simulink model "Lab2_simulink_plc"  (R2023b)
settings: Solver=FixedStepAuto  StartTime=0.0  StopTime=inf  RelTol=1e-3
InitFcn:
  | d13_init.PRE = 50;
undefined symbols (not defined in the file or in its folder): BOOL, DINT, OTL, slplc
libraries: arduinolib x9
(part 4 of 31)

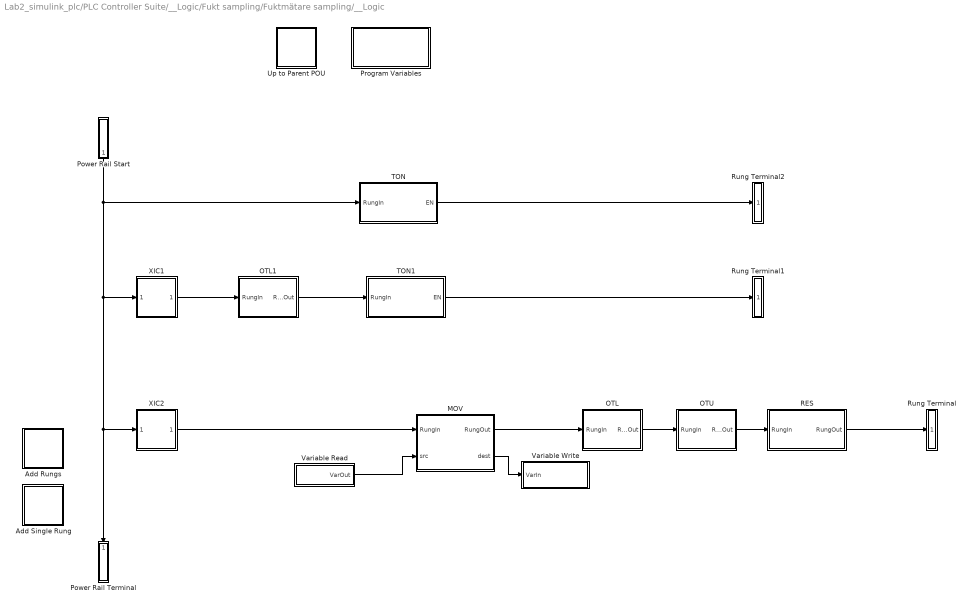
== system Lab2_simulink_plc/PLC Controller Suite/__Logic/Fukt sampling/Fuktmätare sampling/__Logic ==
  Add Rungs : Subsystem  DisableCoverage=on  TreatAsAtomicUnit=on  mask{PLCRungNumberToAdd=2, PLCBlockType=AddRungs}
      mask prompts: PLCRungNumberToAdd "Rung Number:"
      mask Help: web([docroot '/plccoder/ref/addrungs' '.html']);
      mask DialogControl PLCAddRungs Prompt: Add Rungs
      mask DialogControl PLCRungAddButton Prompt: Add
      mask DialogControl PLCRungAddButton Callback: slplc.callbacks.addLadderRungs(gcb);
      mask Display: disp(sprintf(['Add' newline 'Rungs']));
  Add Single Rung : Subsystem  DisableCoverage=on  TreatAsAtomicUnit=on  mask{PLCRungNumberToAdd=1, PLCBlockType=AddSingleRung}
      settings: OpenFcn=slplc.callbacks.addLadderRungs(gcb);
      mask Help: web([docroot '/plccoder/ref/addsinglerung' '.html']);
      mask DialogControl PLCAddRung Prompt: Add Single Rung
      mask Display: disp(sprintf(['Add' newline '1 Rung']));
  MOV : Subsystem  Priority=10  TreatAsAtomicUnit=on  mask{PLCBlockType=MOV, PLCPOUType=stdFC}
      mask Help: web([docroot '/plccoder/ref/mov' '.html']);
      mask DialogControl PLCBlockTitle Prompt: Move (MOV)
      mask DialogControl PLCBlockDesc Prompt: Move a copy of the Source to the Destination. The Source remains unchanged.
      mask Display: disp('MOV');
  OTL : Subsystem  Priority=12  TreatAsAtomicUnit=on  mask{PLCOperandTag=fukt_polling, PLCBlockType=OTL, PLCPOUType=Coil, PLCOperandExpression=xxx_PLC_VAR_fukt_polling}
      mask prompts: PLCOperandTag "Operand Tag:"
      mask init:
      | slplc.callbacks.initInstruction(gcb);
      settings: AttributesFormatString=%<PLCOperandTag>
      mask Help: web([docroot '/plccoder/ref/otl' '.html']);
      mask DialogControl PLCBlockTitle Prompt: Output Latch (OTL)
      mask Display: disp('L');
  OTL1 : Subsystem  Priority=5  TreatAsAtomicUnit=on  mask{PLCOperandTag=fukt_power, PLCBlockType=OTL, PLCPOUType=Coil, PLCOperandExpression=xxx_PLC_VAR_fukt_power}
      mask prompts: PLCOperandTag "Operand Tag:"
      mask init:
      | slplc.callbacks.initInstruction(gcb);
      settings: AttributesFormatString=%<PLCOperandTag>
      mask Help: web([docroot '/plccoder/ref/otl' '.html']);
      mask DialogControl PLCBlockTitle Prompt: Output Latch (OTL)
      mask Display: disp('L');
  OTU : Subsystem  Priority=13  TreatAsAtomicUnit=on  mask{PLCOperandTag=fukt_power, PLCBlockType=OTU, PLCPOUType=Coil, PLCOperandExpression=xxx_PLC_VAR_fukt_power}
      mask prompts: PLCOperandTag "Operand Tag:"
      mask init:
      | slplc.callbacks.initInstruction(gcb);
      settings: AttributesFormatString=%<PLCOperandTag>
      mask Help: web([docroot '/plccoder/ref/otu' '.html']);
      mask DialogControl PLCBlockTitle Prompt: Output Unlatch (OTU)
      mask Display: disp('U');
  Power Rail Start : Subsystem  Priority=1  DisableCoverage=on  TreatAsAtomicUnit=on  mask{PLCBlockType=PowerRailStart}
      mask Help: web([docroot '/plccoder/ref/powerrailstart' '.html']);
      mask DialogControl PLCBlockTitle Prompt: Power Rail Start
      mask DialogControl PLCBlockDesc Prompt: This is the ladder diagram left power rail start block.
  Power Rail Terminal : Subsystem  DisableCoverage=on  TreatAsAtomicUnit=on  mask{PLCBlockType=PowerRailTerminal}
      mask Help: web([docroot '/plccoder/ref/powerrailterminal' '.html']);
      mask DialogControl PLCBlockTitle Prompt: Power Rail Terminal
      mask DialogControl PLCBlockDesc Prompt: This is the ladder diagram left power rail terminal block.
  Program Variables : Subsystem  Tag=ProgramVariableSS  DisableCoverage=on  TreatAsAtomicUnit=on  mask{PLCVarFukt_periodicityName=Fukt_periodicity, PLCVarFukt_periodicityScope=Local, PLCVarFukt_periodicityPortType=Hidden, PLCVarFukt_periodicityPortIndex=6, PLCVarFukt_periodicityDataType=Bus: TIMER, PLCVarFukt_periodicitySize=1, PLCVarFukt_periodicityInitialValue=fukt_period, PLCVarFukt_periodicityToDelete=off, PLCVard13_waitName=d13_wait, PLCVard13_waitScope=Local, PLCVard13_waitPortType=Hidden, PLCVard13_waitPortIndex=6, PLCVard13_waitDataType=Bus: TIMER, PLCVard13_waitSize=1, PLCVard13_waitInitialValue=d13_period, PLCVard13_waitToDelete=off, PLCVarFukt_valueName=Fukt_value, PLCVarFukt_valueScope=External, PLCVarFukt_valuePortType=Hidden, PLCVarFukt_valuePortIndex=6, PLCVarFukt_valueDataType=DINT, PLCVarFukt_valueSize=-1, PLCVarFukt_valueInitialValue=0, PLCVarFukt_valueToDelete=off, PLCVarFukt_value_copyName=Fukt_value_copy, PLCVarFukt_value_copyScope=External, PLCVarFukt_value_copyPortType=Hidden, PLCVarFukt_value_copyPortIndex=6, PLCVarFukt_value_copyDataType=DINT, PLCVarFukt_value_copySize=-1, PLCVarFukt_value_copyInitialValue=0, PLCVarFukt_value_copyToDelete=off, PLCVarfukt_pollingName=fukt_polling, PLCVarfukt_pollingScope=External, PLCVarfukt_pollingPortType=Hidden, PLCVarfukt_pollingPortIndex=6, PLCVarfukt_pollingDataType=DINT, PLCVarfukt_pollingSize=-1, PLCVarfukt_pollingInitialValue=0, PLCVarfukt_pollingToDelete=off, PLCVarfukt_powerName=fukt_power, PLCVarfukt_powerScope=External, PLCVarfukt_powerPortType=Hidden, PLCVarfukt_powerPortIndex=6, PLCVarfukt_powerDataType=DINT, PLCVarfukt_powerSize=-1, PLCVarfukt_powerInitialValue=0, PLCVarfukt_powerToDelete=off}
      mask init:
      | slplc.callbacks.initPOUVarSS(gcb);
      settings: OpenFcn=slplc.callbacks.openProgramVariableSS(gcb);
      mask Help: web([docroot '/plccoder/ref/programvariables' '.html']);
      mask TypeOptions PLCVarFukt_periodicityScope: Local | External
      mask Callback PLCVarFukt_periodicityScope: slplc.callbacks.updatePOUVarSS(gcb);
      mask TypeOptions PLCVarFukt_periodicityPortType: Hidden | Inport | Outport
      mask Callback PLCVarFukt_periodicityPortType: slplc.callbacks.updatePOUVarSS(gcb);
      mask TypeOptions PLCVarFukt_periodicityPortIndex: 1 | 2 | 3 | 4 | 5 | 6
      mask TypeOptions PLCVard13_waitScope: Local | External
      mask Callback PLCVard13_waitScope: slplc.callbacks.updatePOUVarSS(gcb);
      mask TypeOptions PLCVard13_waitPortType: Hidden | Inport | Outport
      mask Callback PLCVard13_waitPortType: slplc.callbacks.updatePOUVarSS(gcb);
      mask TypeOptions PLCVard13_waitPortIndex: 1 | 2 | 3 | 4 | 5 | 6
      mask TypeOptions PLCVarFukt_valueScope: Local | External
      mask Callback PLCVarFukt_valueScope: slplc.callbacks.updatePOUVarSS(gcb);
      mask TypeOptions PLCVarFukt_valuePortType: Hidden | Inport | Outport
      mask Callback PLCVarFukt_valuePortType: slplc.callbacks.updatePOUVarSS(gcb);
      mask TypeOptions PLCVarFukt_valuePortIndex: 1 | 2 | 3 | 4 | 5 | 6
      mask TypeOptions PLCVarFukt_value_copyScope: Local | External
      mask Callback PLCVarFukt_value_copyScope: slplc.callbacks.updatePOUVarSS(gcb);
      mask TypeOptions PLCVarFukt_value_copyPortType: Hidden | Inport | Outport
      mask Callback PLCVarFukt_value_copyPortType: slplc.callbacks.updatePOUVarSS(gcb);
      mask TypeOptions PLCVarFukt_value_copyPortIndex: 1 | 2 | 3 | 4 | 5 | 6
      mask TypeOptions PLCVarfukt_pollingScope: Local | External
      mask Callback PLCVarfukt_pollingScope: slplc.callbacks.updatePOUVarSS(gcb);
      mask TypeOptions PLCVarfukt_pollingPortType: Hidden | Inport | Outport
      mask Callback PLCVarfukt_pollingPortType: slplc.callbacks.updatePOUVarSS(gcb);
      mask TypeOptions PLCVarfukt_pollingPortIndex: 1 | 2 | 3 | 4 | 5 | 6
      mask TypeOptions PLCVarfukt_powerScope: Local | External
      mask Callback PLCVarfukt_powerScope: slplc.callbacks.updatePOUVarSS(gcb);
      mask TypeOptions PLCVarfukt_powerPortType: Hidden | Inport | Outport
      mask Callback PLCVarfukt_powerPortType: slplc.callbacks.updatePOUVarSS(gcb);
      mask TypeOptions PLCVarfukt_powerPortIndex: 1 | 2 | 3 | 4 | 5 | 6
      mask DialogControl PLCBlockTitle Prompt: Program Variable Spreadsheet
      mask DialogControl PLCVarListHeaderName Prompt: Name
      mask DialogControl PLCVarListHeaderScope Prompt: Scope
      mask DialogControl PLCVarListHeaderDataType Prompt: Data Type
      mask DialogControl PLCVarListHeaderSize Prompt: Size
      mask DialogControl PLCVarListHeaderInitialValue Prompt: Initial Value
      mask DialogControl PLCVarListHeaderToDelete Prompt: Delete
  RES : Subsystem  Tag=B  Priority=14  TreatAsAtomicUnit=on  mask{PLCOperandTag=Fukt_periodicity, PLCTagDataType=TIMER, PLCBlockType=RES, PLCPOUType=stdFC, PLCOperandExpression=xxx_PLC_VAR_Fukt_periodicity}
      mask prompts: PLCOperandTag "Operand Tag To Reset:"; PLCTagDataType "Tag Structure:"
      mask init:
      | block = gcb;
      | maskObj = Simulink.Mask.get(block);
      | maskObj.Display = sprintf('disp([''%s''  newline newline ''%s'']);', 'RES', PLCOperandTag);
      | slplc.callbacks.initResetBlock(block);
      | slplc.callbacks.initInstruction(block);
      settings: AttributesFormatString=%<PLCBlockType>: %<PLCOperandTag>
      mask Help: web([docroot '/plccoder/ref/res' '.html']);
      mask TypeOptions PLCTagDataType: TIMER | COUNTER | CONTROL
      mask DialogControl PLCBlockTitle Prompt: Reset TIMER, COUNTER, or CONTROL structure (RES)
      mask Display: disp(['RES'  newline newline 'Fukt_periodicity']);
  Rung Terminal : Subsystem  Priority=15  DisableCoverage=on  TreatAsAtomicUnit=on  mask{PLCRungTerminalIndex=3, PLCBlockType=RungTerminal}
      settings: AttributesFormatString=Rung %<PLCRungTerminalIndex>
      mask Help: web([docroot '/plccoder/ref/rungterminal' '.html']);
      mask DialogControl PLCBlockTitle Prompt: Rung Terminal
      mask DialogControl PLCBlockDescText Prompt: This is the ladder diagram rung terminal.
  Rung Terminal1 : Subsystem  Priority=7  DisableCoverage=on  TreatAsAtomicUnit=on  mask{PLCRungTerminalIndex=2, PLCBlockType=RungTerminal}
      settings: AttributesFormatString=Rung %<PLCRungTerminalIndex>
      mask Help: web([docroot '/plccoder/ref/rungterminal' '.html']);
      mask DialogControl PLCBlockTitle Prompt: Rung Terminal
      mask DialogControl PLCBlockDescText Prompt: This is the ladder diagram rung terminal.
  Rung Terminal2 : Subsystem  Priority=3  DisableCoverage=on  TreatAsAtomicUnit=on  mask{PLCRungTerminalIndex=1, PLCBlockType=RungTerminal}
      settings: AttributesFormatString=Rung %<PLCRungTerminalIndex>
      mask Help: web([docroot '/plccoder/ref/rungterminal' '.html']);
      mask DialogControl PLCBlockTitle Prompt: Rung Terminal
      mask DialogControl PLCBlockDescText Prompt: This is the ladder diagram rung terminal.
  TON : Subsystem  Priority=2  TreatAsAtomicUnit=on  mask{PLCOperandTag=Fukt_periodicity, PLCTimerSampleTime=0.2, PLCBlockType=TON, PLCPOUType=stdFB, PLCOperandAccess=ReadWrite, PLCOperandExpression=xxx_PLC_VAR_Fukt_periodicity}
      mask prompts: PLCOperandTag "Timer Tag:"; PLCTimerSampleTime "Timer Sample Time:"
      mask init:
      | block = gcb;
      | maskObj = Simulink.Mask.get(block);
      | maskObj.Display = sprintf('disp([''%s'' newline newline ''%s'']);', PLCBlockType, PLCOperandTag);
      | slplc.callbacks.initInstruction(block);
      settings: AttributesFormatString=%<PLCBlockType>: %<PLCOperandTag>
      mask Help: web([docroot '/plccoder/ref/ton' '.html']);
      mask DialogControl PLCBlockTitle Prompt: Timer On Delay (TON)
      mask Display: disp(['TON' newline newline 'Fukt_periodicity']);
  TON1 : Subsystem  Priority=6  TreatAsAtomicUnit=on  mask{PLCOperandTag=d13_wait, PLCTimerSampleTime=0.2, PLCBlockType=TON, PLCPOUType=stdFB, PLCOperandAccess=ReadWrite, PLCOperandExpression=xxx_PLC_VAR_d13_wait}
      mask prompts: PLCOperandTag "Timer Tag:"; PLCTimerSampleTime "Timer Sample Time:"
      mask init:
      | block = gcb;
      | maskObj = Simulink.Mask.get(block);
      | maskObj.Display = sprintf('disp([''%s'' newline newline ''%s'']);', PLCBlockType, PLCOperandTag);
      | slplc.callbacks.initInstruction(block);
      settings: AttributesFormatString=%<PLCBlockType>: %<PLCOperandTag>
      mask Help: web([docroot '/plccoder/ref/ton' '.html']);
      mask DialogControl PLCBlockTitle Prompt: Timer On Delay (TON)
      mask Display: disp(['TON' newline newline 'd13_wait']);
  Up to Parent POU : Subsystem  DisableCoverage=on  TreatAsAtomicUnit=on  mask{PLCBlockType=OpenParentPOU}
      settings: OpenFcn=slplc.callbacks.openParentPOUBlock(gcb);
      mask Help: web([docroot '/plccoder/ref/uptoparentpou' '.html']);
      mask DialogControl PLCBlockTitle Prompt: Add Single Rung
  Variable Read : Subsystem  Priority=9  TreatAsAtomicUnit=on  mask{PLCOperandTag=Fukt_value, PLCBlockType=VariableRead, PLCOperandAccess=Read, PLCPOUType=VariableRead, PLCOperandExpression=xxx_PLC_VAR_Fukt_value}
      mask prompts: PLCOperandTag "Data Tag:"
      mask init:
      | block = gcb;
      | maskObj = Simulink.Mask.get(block);
      | maskObj.Display = sprintf('disp(''%s'');', PLCOperandTag);
      | slplc.callbacks.initInstruction(block);
      mask Help: web([docroot '/plccoder/ref/variableread' '.html']);
      mask DialogControl PLCBlockTitle Prompt: Data Tag Read
      mask Display: disp('Fukt_value');
  Variable Write : Subsystem  Priority=11  TreatAsAtomicUnit=on  mask{PLCOperandTag=Fukt_value_copy, PLCBlockType=VariableWrite, PLCOperandAccess=Write, PLCPOUType=VariableWrite, PLCOperandExpression=xxx_PLC_VAR_Fukt_value_copy}
      mask prompts: PLCOperandTag "Data Tag:"
      mask init: same as Lab2_simulink_plc/PLC Controller Suite/__Logic/Fukt logic/Program/__Logic/Variable Write
      mask Help: web([docroot '/plccoder/ref/variablewrite' '.html']);
      mask DialogControl PLCBlockTitle Prompt: Data Tag Write
      mask Display: disp('Fukt_value_copy');
  XIC1 : Subsystem  Priority=4  TreatAsAtomicUnit=on  mask{PLCOperandTag=Fukt_periodicity.DN, PLCBlockType=XIC, PLCPOUType=Contact, PLCOperandExpression=xxx_PLC_VAR_Fukt_periodicity.DN}
      mask prompts: PLCOperandTag "Operand Tag:"
      mask init:
      | slplc.callbacks.initInstruction(gcb);
      settings: AttributesFormatString=%<PLCOperandTag>
      mask Help: web([docroot '/plccoder/ref/xic' '.html']);
      mask DialogControl PLCBlockTitle Prompt: Examine If Closed (XIC)
  XIC2 : Subsystem  Priority=8  TreatAsAtomicUnit=on  mask{PLCOperandTag=d13_wait.DN, PLCBlockType=XIC, PLCPOUType=Contact, PLCOperandExpression=xxx_PLC_VAR_d13_wait.DN}
      mask prompts: PLCOperandTag "Operand Tag:"
      mask init:
      | slplc.callbacks.initInstruction(gcb);
      settings: AttributesFormatString=%<PLCOperandTag>
      mask Help: web([docroot '/plccoder/ref/xic' '.html']);
      mask DialogControl PLCBlockTitle Prompt: Examine If Closed (XIC)
  wires:
  Variable Read/1(VarOut) -> MOV/2(src)
  MOV/1(RungOut) -> OTL/1(RungIn)
  MOV/2(dest) -> Variable Write/1(VarIn)
  TON/1(EN) -> Rung Terminal2/1(RungIn)
  XIC1/1(RungOut) -> OTL1/1(RungIn)
  XIC2/1(RungOut) -> MOV/1(RungIn)
  OTL1/1(RungOut) -> TON1/1(RungIn)
  OTL/1(RungOut) -> OTU/1(RungIn)
  Power Rail Start/1(PowerOut) -> TON/1(RungIn), XIC1/1(RungIn), XIC2/1(RungIn), Power Rail Terminal/1(PowerIn)
  TON1/1(EN) -> Rung Terminal1/1(RungIn)
  OTU/1(RungOut) -> RES/1(RungIn)
  RES/1(RungOut) -> Rung Terminal/1(RungIn)
